simulink model "CCMMotor"  (R2022b) (continued)
== system CCMMotor == (continued)
  AirFlapPosnFrntReqForRowFirstForActrFlrLe_AirFlapPosnFrntReqForRowFirstForActrFlrLe/1 -> CCMMotor_Runnable_sys/2(AirFlapPosnFrntReqForRowFirstForActrFlrLe_AirFlapPosnFrntReqForRowFirstForActrFlrLe_read)
  AirFlapPosnFrntReqForRowFirstForActrFlrRi_AirFlapPosnFrntReqForRowFirstForActrFlrRi/1 -> CCMMotor_Runnable_sys/3(AirFlapPosnFrntReqForRowFirstForActrFlrRi_AirFlapPosnFrntReqForRowFirstForActrFlrRi_read)
  AirFlapPosnFrntReqForRowFirstForActrVentLe_AirFlapPosnFrntReqForRowFirstForActrVentLe/1 -> CCMMotor_Runnable_sys/4(AirFlapPosnFrntReqForRowFirstForActrVentLe_AirFlapPosnFrntReqForRowFirstForActrVentLe_read)
  AirFlapPosnFrntReqForRowFirstForActrVentRi_AirFlapPosnFrntReqForRowFirstForActrVentRi/1 -> CCMMotor_Runnable_sys/5(AirFlapPosnFrntReqForRowFirstForActrVentRi_AirFlapPosnFrntReqForRowFirstForActrVentRi_read)
  AirFlapPosnFrntReqForRowFirstForActrVentSideLe_AirFlapPosnFrntReqForRowFirstForActrVentSideLe/1 -> CCMMotor_Runnable_sys/6(AirFlapPosnFrntReqForRowFirstForActrVentSideLe_AirFlapPosnFrntReqForRowFirstForActrVentSideLe_read)
  AirFlapPosnFrntReqForRowFirstForActrVentSideRi_AirFlapPosnFrntReqForRowFirstForActrVentSideRi/1 -> CCMMotor_Runnable_sys/7(AirFlapPosnFrntReqForRowFirstForActrVentSideRi_AirFlapPosnFrntReqForRowFirstForActrVentSideRi_read)
  AirFlapPosnFrntReqForRowFirstForOsa_AirFlapPosnFrntReqForRowFirstForOsa/1 -> CCMMotor_Runnable_sys/8(AirFlapPosnFrntReqForRowFirstForOsa_AirFlapPosnFrntReqForRowFirstForOsa_read)
  AirFlapPosnFrntReqForRowFirstForRecirc_AirFlapPosnFrntReqForRowFirstForRecirc/1 -> CCMMotor_Runnable_sys/9(AirFlapPosnFrntReqForRowFirstForRecirc_AirFlapPosnFrntReqForRowFirstForRecirc_read)
  AirFlapPosnFrntReqForRowSecForActrTRi_AirFlapPosnFrntReqForRowSecForActrTRi/1 -> CCMMotor_Runnable_sys/10(AirFlapPosnFrntReqForRowSecForActrTRi_AirFlapPosnFrntReqForRowSecForActrTRi_read)
  PwrMdAllMtrSlp_PwrMdAllMtrSlp/1 -> CCMMotor_Runnable_sys/11(PwrMdAllMtrSlp_PwrMdAllMtrSlp_read)
  SysServCarCfgSts_SysServCarID/1 -> CCMMotor_Runnable_sys/12(SysServCarCfgSts_SysServCarID_read)
  SysServCarCfgSts_SysServCCPAQSSts/1 -> CCMMotor_Runnable_sys/13(SysServCarCfgSts_SysServCCPAQSSts_read)
  SysServCarCfgSts_SysServCCPHEXSensorSts/1 -> CCMMotor_Runnable_sys/14(SysServCarCfgSts_SysServCCPHEXSensorSts_read)
  SysServCarCfgSts_SysServCCPLin2AFUSts/1 -> CCMMotor_Runnable_sys/15(SysServCarCfgSts_SysServCCPLin2AFUSts_read)
  SysServCarCfgSts_SysServCCPLin2AIUSts/1 -> CCMMotor_Runnable_sys/16(SysServCarCfgSts_SysServCCPLin2AIUSts_read)
  SysServCarCfgSts_SysServCCPLin2CDSSts/1 -> CCMMotor_Runnable_sys/17(SysServCarCfgSts_SysServCCPLin2CDSSts_read)
  SysServCarCfgSts_SysServCCPLin2PMSISts/1 -> CCMMotor_Runnable_sys/18(SysServCarCfgSts_SysServCCPLin2PMSISts_read)
  SysServCarCfgSts_SysServCCPLin3VGASts/1 -> CCMMotor_Runnable_sys/19(SysServCarCfgSts_SysServCCPLin3VGASts_read)
  SysServCarCfgSts_SysServDUAirVentSnrSts/1 -> CCMMotor_Runnable_sys/20(SysServCarCfgSts_SysServDUAirVentSnrSts_read)
  SysServCarCfgSts_SysServDUBlowerPwmSignSts/1 -> CCMMotor_Runnable_sys/21(SysServCarCfgSts_SysServDUBlowerPwmSignSts_read)
  SysServCarCfgSts_SysServDUBlowerPwmSts/1 -> CCMMotor_Runnable_sys/22(SysServCarCfgSts_SysServDUBlowerPwmSts_read)
  SysServCarCfgSts_SysServDUDCDefrostSts/1 -> CCMMotor_Runnable_sys/23(SysServCarCfgSts_SysServDUDCDefrostSts_read)
  SysServCarCfgSts_SysServDUDCModeSts/1 -> CCMMotor_Runnable_sys/24(SysServCarCfgSts_SysServDUDCModeSts_read)
  SysServCarCfgSts_SysServDUDCRecSts/1 -> CCMMotor_Runnable_sys/25(SysServCarCfgSts_SysServDUDCRecSts_read)
  SysServCarCfgSts_SysServDUDCSModeSts/1 -> CCMMotor_Runnable_sys/26(SysServCarCfgSts_SysServDUDCSModeSts_read)
  SysServCarCfgSts_SysServDUDCSTLSts/1 -> CCMMotor_Runnable_sys/27(SysServCarCfgSts_SysServDUDCSTLSts_read)
  SysServCarCfgSts_SysServDUDCTLSts/1 -> CCMMotor_Runnable_sys/28(SysServCarCfgSts_SysServDUDCTLSts_read)
  SysServCarCfgSts_SysServDUDCTRSts/1 -> CCMMotor_Runnable_sys/29(SysServCarCfgSts_SysServDUDCTRSts_read)
  SysServCarCfgSts_SysServDUFLFootSts/1 -> CCMMotor_Runnable_sys/30(SysServCarCfgSts_SysServDUFLFootSts_read)
  SysServCarCfgSts_SysServDUFLVentSts/1 -> CCMMotor_Runnable_sys/31(SysServCarCfgSts_SysServDUFLVentSts_read)
  SysServCarCfgSts_SysServDUFRFootSts/1 -> CCMMotor_Runnable_sys/32(SysServCarCfgSts_SysServDUFRFootSts_read)
  SysServCarCfgSts_SysServDUFRVentSts/1 -> CCMMotor_Runnable_sys/33(SysServCarCfgSts_SysServDUFRVentSts_read)
  SysServCarCfgSts_SysServDUIncarMotorSts/1 -> CCMMotor_Runnable_sys/34(SysServCarCfgSts_SysServDUIncarMotorSts_read)
  SysServCarCfgSts_SysServDULin1HBMFSts/1 -> CCMMotor_Runnable_sys/35(SysServCarCfgSts_SysServDULin1HBMFSts_read)
  SysServCarCfgSts_SysServDULin4HFAFSts/1 -> CCMMotor_Runnable_sys/36(SysServCarCfgSts_SysServDULin4HFAFSts_read)
  SysServCarCfgSts_SysServDUSFLFootSts/1 -> CCMMotor_Runnable_sys/37(SysServCarCfgSts_SysServDUSFLFootSts_read)
  SysServCarCfgSts_SysServDUSFLVentSts/1 -> CCMMotor_Runnable_sys/38(SysServCarCfgSts_SysServDUSFLVentSts_read)
  SysServCarCfgSts_SysServDUSFRFootSts/1 -> CCMMotor_Runnable_sys/39(SysServCarCfgSts_SysServDUSFRFootSts_read)
  SysServCarCfgSts_SysServDUSFRVentSts/1 -> CCMMotor_Runnable_sys/40(SysServCarCfgSts_SysServDUSFRVentSts_read)
  SysServCarCfgSts_SysServDUSnsrForFCmptmtTSts/1 -> CCMMotor_Runnable_sys/41(SysServCarCfgSts_SysServDUSnsrForFCmptmtTSts_read)
  SysServCarCfgSts_SysServDUSnsrForRair/1 -> CCMMotor_Runnable_sys/42(SysServCarCfgSts_SysServDUSnsrForRair_read)
  SysServCarCfgSts_SysServDUSnsrForRCmptmtTSts/1 -> CCMMotor_Runnable_sys/43(SysServCarCfgSts_SysServDUSnsrForRCmptmtTSts_read)
  VehModMngtGlbSafe1_VehModMngtGlbSafe1/1 -> CCMMotor_Runnable_sys/44(VehModMngtGlbSafe1_VehModMngtGlbSafe1_read)
  ReqForActrCal_ReqForActrCal/1 -> CCMMotor_Runnable_sys/45(ReqForActrCal_ReqForActrCal_read)
  ActrFrntReqForRowFirstLeModDistbn_ActrFrntReqForRowFirstLeModDistbn/1 -> CCMMotor_Runnable_sys/46(ActrFrntReqForRowFirstLeModDistbn_ActrFrntReqForRowFirstLeModDistbn_read)
  ActrFrntReqForRowFirstLeModDistbn_ActrFrntReqForRowFirstLeModDistbn_IsUpdated/1 -> CCMMotor_Runnable_sys/47(ActrFrntReqForRowFirstLeModDistbn_ActrFrntReqForRowFirstLeModDistbn_IsUpdated_read)
  ActrFrntReqForRowFirstModDistbn_ActrFrntReqForRowFirstModDistbn/1 -> CCMMotor_Runnable_sys/48(ActrFrntReqForRowFirstModDistbn_ActrFrntReqForRowFirstModDistbn_read)
  ActrFrntReqForRowFirstModDistbn_ActrFrntReqForRowFirstModDistbn_IsUpdated/1 -> CCMMotor_Runnable_sys/49(ActrFrntReqForRowFirstModDistbn_ActrFrntReqForRowFirstModDistbn_IsUpdated_read)
  ActrFrntReqForRowSecModDistbnLe_ActrFrntReqForRowSecModDistbnLe/1 -> CCMMotor_Runnable_sys/50(ActrFrntReqForRowSecModDistbnLe_ActrFrntReqForRowSecModDistbnLe_read)
  ActrFrntReqForRowSecModDistbnLe_ActrFrntReqForRowSecModDistbnLe_IsUpdated/1 -> CCMMotor_Runnable_sys/51(ActrFrntReqForRowSecModDistbnLe_ActrFrntReqForRowSecModDistbnLe_IsUpdated_read)
  ActrPosnFrnReqForOsaAndRecirc_ActrPosnFrnReqForOsaAndRecirc/1 -> CCMMotor_Runnable_sys/52(ActrPosnFrnReqForOsaAndRecirc_ActrPosnFrnReqForOsaAndRecirc_read)
  ActrPosnFrnReqForOsaAndRecirc_ActrPosnFrnReqForOsaAndRecirc_IsUpdated/1 -> CCMMotor_Runnable_sys/53(ActrPosnFrnReqForOsaAndRecirc_ActrPosnFrnReqForOsaAndRecirc_IsUpdated_read)
  ActrReqForModDistbnRe_ActrReqForModDistbnRe/1 -> CCMMotor_Runnable_sys/54(ActrReqForModDistbnRe_ActrReqForModDistbnRe_read)
  ActrReqForModDistbnRe_ActrReqForModDistbnRe_IsUpdated/1 -> CCMMotor_Runnable_sys/55(ActrReqForModDistbnRe_ActrReqForModDistbnRe_IsUpdated_read)
  AirFlapPosnFrntReqForRowFirstForActrDefrst_AirFlapPosnFrntReqForRowFirstForActrDefrst/1 -> CCMMotor_Runnable_sys/56(AirFlapPosnFrntReqForRowFirstForActrDefrst_AirFlapPosnFrntReqForRowFirstForActrDefrst_read)
  AirFlapPosnFrntReqForRowFirstForActrDefrst_AirFlapPosnFrntReqForRowFirstForActrDefrst_IsUpdated/1 -> CCMMotor_Runnable_sys/57(AirFlapPosnFrntReqForRowFirstForActrDefrst_AirFlapPosnFrntReqForRowFirstForActrDefrst_IsUpdated_read)
  AirFlapPosnFrntReqForRowFirstForActrTLe_AirFlapPosnFrntReqForRowFirstForActrTLe/1 -> CCMMotor_Runnable_sys/58(AirFlapPosnFrntReqForRowFirstForActrTLe_AirFlapPosnFrntReqForRowFirstForActrTLe_read)
  AirFlapPosnFrntReqForRowFirstForActrTLe_AirFlapPosnFrntReqForRowFirstForActrTLe_IsUpdated/1 -> CCMMotor_Runnable_sys/59(AirFlapPosnFrntReqForRowFirstForActrTLe_AirFlapPosnFrntReqForRowFirstForActrTLe_IsUpdated_read)
  AirFlapPosnFrntReqForRowFirstForActrTRi_AirFlapPosnFrntReqForRowFirstForActrTRi/1 -> CCMMotor_Runnable_sys/60(AirFlapPosnFrntReqForRowFirstForActrTRi_AirFlapPosnFrntReqForRowFirstForActrTRi_read)
  AirFlapPosnFrntReqForRowFirstForActrTRi_AirFlapPosnFrntReqForRowFirstForActrTRi_IsUpdated/1 -> CCMMotor_Runnable_sys/61(AirFlapPosnFrntReqForRowFirstForActrTRi_AirFlapPosnFrntReqForRowFirstForActrTRi_IsUpdated_read)
  AirFlapPosnFrntReqForRowSecForActrTLe_AirFlapPosnFrntReqForRowSecForActrTLe/1 -> CCMMotor_Runnable_sys/62(AirFlapPosnFrntReqForRowSecForActrTLe_AirFlapPosnFrntReqForRowSecForActrTLe_read)
  AirFlapPosnFrntReqForRowSecForActrTLe_AirFlapPosnFrntReqForRowSecForActrTLe_IsUpdated/1 -> CCMMotor_Runnable_sys/63(AirFlapPosnFrntReqForRowSecForActrTLe_AirFlapPosnFrntReqForRowSecForActrTLe_IsUpdated_read)
  CarCfgForClimaCtrl_CarCfgForAirClng/1 -> CCMMotor_Runnable_sys/64(CarCfgForClimaCtrl_CarCfgForAirClng_read)
  CarCfgForClimaCtrl_CarCfgForAirClnrAdv/1 -> CCMMotor_Runnable_sys/65(CarCfgForClimaCtrl_CarCfgForAirClnrAdv_read)
  CarCfgForClimaCtrl_CarCfgForAirFrag/1 -> CCMMotor_Runnable_sys/66(CarCfgForClimaCtrl_CarCfgForAirFrag_read)
  CarCfgForClimaCtrl_CarCfgForAirMtrlSnsr/1 -> CCMMotor_Runnable_sys/67(CarCfgForClimaCtrl_CarCfgForAirMtrlSnsr_read)
  CarCfgForClimaCtrl_CarCfgForAirQly/1 -> CCMMotor_Runnable_sys/68(CarCfgForClimaCtrl_CarCfgForAirQly_read)
  CarCfgForClimaCtrl_CarCfgForAmntOfSeat/1 -> CCMMotor_Runnable_sys/69(CarCfgForClimaCtrl_CarCfgForAmntOfSeat_read)
  CarCfgForClimaCtrl_CarCfgForAutDemst/1 -> CCMMotor_Runnable_sys/70(CarCfgForClimaCtrl_CarCfgForAutDemst_read)
  CarCfgForClimaCtrl_CarCfgForAuxWtrPmp/1 -> CCMMotor_Runnable_sys/71(CarCfgForClimaCtrl_CarCfgForAuxWtrPmp_read)
  CarCfgForClimaCtrl_CarCfgForBRAND/1 -> CCMMotor_Runnable_sys/72(CarCfgForClimaCtrl_CarCfgForBRAND_read)
  CarCfgForClimaCtrl_CarCfgForBypVlvInHvac/1 -> CCMMotor_Runnable_sys/73(CarCfgForClimaCtrl_CarCfgForBypVlvInHvac_read)
  CarCfgForClimaCtrl_CarCfgForClsVlvInHvac/1 -> CCMMotor_Runnable_sys/74(CarCfgForClimaCtrl_CarCfgForClsVlvInHvac_read)
  CarCfgForClimaCtrl_CarCfgForCmprAcTyp/1 -> CCMMotor_Runnable_sys/75(CarCfgForClimaCtrl_CarCfgForCmprAcTyp_read)
  CarCfgForClimaCtrl_CarCfgForCmptmtClng/1 -> CCMMotor_Runnable_sys/76(CarCfgForClimaCtrl_CarCfgForCmptmtClng_read)
  CarCfgForClimaCtrl_CarCfgForElectricLiftingModule/1 -> CCMMotor_Runnable_sys/77(CarCfgForClimaCtrl_CarCfgForElectricLiftingModule_read)
  CarCfgForClimaCtrl_CarCfgForElecVentAdjmt/1 -> CCMMotor_Runnable_sys/78(CarCfgForClimaCtrl_CarCfgForElecVentAdjmt_read)
  CarCfgForClimaCtrl_CarCfgForExtVentnClimaCtrl/1 -> CCMMotor_Runnable_sys/79(CarCfgForClimaCtrl_CarCfgForExtVentnClimaCtrl_read)
  CarCfgForClimaCtrl_CarCfgForFlcTyp/1 -> CCMMotor_Runnable_sys/80(CarCfgForClimaCtrl_CarCfgForFlcTyp_read)
  CarCfgForClimaCtrl_CarCfgForFoldCSD/1 -> CCMMotor_Runnable_sys/81(CarCfgForClimaCtrl_CarCfgForFoldCSD_read)
  CarCfgForClimaCtrl_CarCfgForFuTyp/1 -> CCMMotor_Runnable_sys/82(CarCfgForClimaCtrl_CarCfgForFuTyp_read)
  CarCfgForClimaCtrl_CarCfgForGlassTyp/1 -> CCMMotor_Runnable_sys/83(CarCfgForClimaCtrl_CarCfgForGlassTyp_read)
  CarCfgForClimaCtrl_CarCfgForHeadRadar/1 -> CCMMotor_Runnable_sys/84(CarCfgForClimaCtrl_CarCfgForHeadRadar_read)
  CarCfgForClimaCtrl_CarCfgForHeatPmp/1 -> CCMMotor_Runnable_sys/85(CarCfgForClimaCtrl_CarCfgForHeatPmp_read)
  CarCfgForClimaCtrl_CarCfgForHeatrDurgDrvg/1 -> CCMMotor_Runnable_sys/86(CarCfgForClimaCtrl_CarCfgForHeatrDurgDrvg_read)
  CarCfgForClimaCtrl_CarCfgForHeatrTyp/1 -> CCMMotor_Runnable_sys/87(CarCfgForClimaCtrl_CarCfgForHeatrTyp_read)
  CarCfgForClimaCtrl_CarCfgForHvacFilTyp/1 -> CCMMotor_Runnable_sys/88(CarCfgForClimaCtrl_CarCfgForHvacFilTyp_read)
  CarCfgForClimaCtrl_CarCfgForHvacFlrSnsrForT/1 -> CCMMotor_Runnable_sys/89(CarCfgForClimaCtrl_CarCfgForHvacFlrSnsrForT_read)
  CarCfgForClimaCtrl_CarCfgForHvacHeatrSnsrT/1 -> CCMMotor_Runnable_sys/90(CarCfgForClimaCtrl_CarCfgForHvacHeatrSnsrT_read)
  CarCfgForClimaCtrl_CarCfgForHvacRe/1 -> CCMMotor_Runnable_sys/91(CarCfgForClimaCtrl_CarCfgForHvacRe_read)
  CarCfgForClimaCtrl_CarCfgForHvacTyp/1 -> CCMMotor_Runnable_sys/92(CarCfgForClimaCtrl_CarCfgForHvacTyp_read)
  CarCfgForClimaCtrl_CarCfgForInteriorViewCamera/1 -> CCMMotor_Runnable_sys/93(CarCfgForClimaCtrl_CarCfgForInteriorViewCamera_read)
  CarCfgForClimaCtrl_CarCfgForLifeDetection/1 -> CCMMotor_Runnable_sys/94(CarCfgForClimaCtrl_CarCfgForLifeDetection_read)
  CarCfgForClimaCtrl_CarCfgForLoVoltElecCfg/1 -> CCMMotor_Runnable_sys/95(CarCfgForClimaCtrl_CarCfgForLoVoltElecCfg_read)
  CarCfgForClimaCtrl_CarCfgForNavSysandIntnmap/1 -> CCMMotor_Runnable_sys/96(CarCfgForClimaCtrl_CarCfgForNavSysandIntnmap_read)
  CarCfgForClimaCtrl_CarCfgForNrOfDoor/1 -> CCMMotor_Runnable_sys/97(CarCfgForClimaCtrl_CarCfgForNrOfDoor_read)
  CarCfgForClimaCtrl_CarCfgForPipeInFuTank/1 -> CCMMotor_Runnable_sys/98(CarCfgForClimaCtrl_CarCfgForPipeInFuTank_read)
  CarCfgForClimaCtrl_CarCfgForPowerSeats/1 -> CCMMotor_Runnable_sys/99(CarCfgForClimaCtrl_CarCfgForPowerSeats_read)
  CarCfgForClimaCtrl_CarCfgForPreClng/1 -> CCMMotor_Runnable_sys/100(CarCfgForClimaCtrl_CarCfgForPreClng_read)
  CarCfgForClimaCtrl_CarCfgForPrkgCDSNotAvl/1 -> CCMMotor_Runnable_sys/101(CarCfgForClimaCtrl_CarCfgForPrkgCDSNotAvl_read)
  CarCfgForClimaCtrl_CarCfgForPrkgClima/1 -> CCMMotor_Runnable_sys/102(CarCfgForClimaCtrl_CarCfgForPrkgClima_read)
  CarCfgForClimaCtrl_CarCfgForPrpsnTyp/1 -> CCMMotor_Runnable_sys/103(CarCfgForClimaCtrl_CarCfgForPrpsnTyp_read)
  CarCfgForClimaCtrl_CarCfgForPTCInFrontHVAC/1 -> CCMMotor_Runnable_sys/104(CarCfgForClimaCtrl_CarCfgForPTCInFrontHVAC_read)
  CarCfgForClimaCtrl_CarCfgForRemEngStrtPreCdn/1 -> CCMMotor_Runnable_sys/105(CarCfgForClimaCtrl_CarCfgForRemEngStrtPreCdn_read)
  CarCfgForClimaCtrl_CarCfgForRoofHatch/1 -> CCMMotor_Runnable_sys/106(CarCfgForClimaCtrl_CarCfgForRoofHatch_read)
  CarCfgForClimaCtrl_CarCfgForSeatHeatgCal/1 -> CCMMotor_Runnable_sys/107(CarCfgForClimaCtrl_CarCfgForSeatHeatgCal_read)
  CarCfgForClimaCtrl_CarCfgForSeatHeatgForRowFirst/1 -> CCMMotor_Runnable_sys/108(CarCfgForClimaCtrl_CarCfgForSeatHeatgForRowFirst_read)
  CarCfgForClimaCtrl_CarCfgForSeatHeatgForRowSec/1 -> CCMMotor_Runnable_sys/109(CarCfgForClimaCtrl_CarCfgForSeatHeatgForRowSec_read)
  CarCfgForClimaCtrl_CarCfgForSeatVentnForRowFirst/1 -> CCMMotor_Runnable_sys/110(CarCfgForClimaCtrl_CarCfgForSeatVentnForRowFirst_read)
  CarCfgForClimaCtrl_CarCfgForSeatVentnForRowSec/1 -> CCMMotor_Runnable_sys/111(CarCfgForClimaCtrl_CarCfgForSeatVentnForRowSec_read)
  CarCfgForClimaCtrl_CarCfgForSecRowVentnCenter/1 -> CCMMotor_Runnable_sys/112(CarCfgForClimaCtrl_CarCfgForSecRowVentnCenter_read)
  CarCfgForClimaCtrl_CarCfgForSecRowVentnSide/1 -> CCMMotor_Runnable_sys/113(CarCfgForClimaCtrl_CarCfgForSecRowVentnSide_read)
  CarCfgForClimaCtrl_CarCfgForSmallFlowCyc/1 -> CCMMotor_Runnable_sys/114(CarCfgForClimaCtrl_CarCfgForSmallFlowCyc_read)
  CarCfgForClimaCtrl_CarCfgForSmkSnsr/1 -> CCMMotor_Runnable_sys/115(CarCfgForClimaCtrl_CarCfgForSmkSnsr_read)
  CarCfgForClimaCtrl_CarCfgForSnsrForCmptmtT/1 -> CCMMotor_Runnable_sys/116(CarCfgForClimaCtrl_CarCfgForSnsrForCmptmtT_read)
  CarCfgForClimaCtrl_CarCfgForSnsrHumPrsnt/1 -> CCMMotor_Runnable_sys/117(CarCfgForClimaCtrl_CarCfgForSnsrHumPrsnt_read)
  CarCfgForClimaCtrl_CarCfgForStartDevice/1 -> CCMMotor_Runnable_sys/118(CarCfgForClimaCtrl_CarCfgForStartDevice_read)
  CarCfgForClimaCtrl_CarCfgForSteerWhlHeatg/1 -> CCMMotor_Runnable_sys/119(CarCfgForClimaCtrl_CarCfgForSteerWhlHeatg_read)
  CarCfgForClimaCtrl_CarCfgForSteerWhlPosn/1 -> CCMMotor_Runnable_sys/120(CarCfgForClimaCtrl_CarCfgForSteerWhlPosn_read)
  CarCfgForClimaCtrl_CarCfgForSunSnsr/1 -> CCMMotor_Runnable_sys/121(CarCfgForClimaCtrl_CarCfgForSunSnsr_read)
  CarCfgForClimaCtrl_CarCfgForTelmNodTyp/1 -> CCMMotor_Runnable_sys/122(CarCfgForClimaCtrl_CarCfgForTelmNodTyp_read)
  CarCfgForClimaCtrl_CarCfgForTEMPEREDSTORAGEBOX/1 -> CCMMotor_Runnable_sys/123(CarCfgForClimaCtrl_CarCfgForTEMPEREDSTORAGEBOX_read)
  CarCfgForClimaCtrl_CarCfgForThermMngt/1 -> CCMMotor_Runnable_sys/124(CarCfgForClimaCtrl_CarCfgForThermMngt_read)
  CarCfgForClimaCtrl_CarCfgForVehTyp/1 -> CCMMotor_Runnable_sys/125(CarCfgForClimaCtrl_CarCfgForVehTyp_read)
  CarCfgForClimaCtrl_CarCfgForVentLiftMotorExiSts/1 -> CCMMotor_Runnable_sys/126(CarCfgForClimaCtrl_CarCfgForVentLiftMotorExiSts_read)
  CarCfgForClimaCtrl_CarCfgForVgaMotorExistSts/1 -> CCMMotor_Runnable_sys/127(CarCfgForClimaCtrl_CarCfgForVgaMotorExistSts_read)
  CarCfgForClimaCtrl_CarCfgForWadingAssistSystem/1 -> CCMMotor_Runnable_sys/128(CarCfgForClimaCtrl_CarCfgForWadingAssistSystem_read)
  CarCfgForClimaCtrl_CarCfgForWindHeatd/1 -> CCMMotor_Runnable_sys/129(CarCfgForClimaCtrl_CarCfgForWindHeatd_read)
  CarCfgForClimaCtrl_CarCfgWindFollowHumanMov/1 -> CCMMotor_Runnable_sys/130(CarCfgForClimaCtrl_CarCfgWindFollowHumanMov_read)
  SysServCarCfgSts_SysServCCPBGMNum/1 -> CCMMotor_Runnable_sys/131(SysServCarCfgSts_SysServCCPBGMNum_read)
  SysServCarCfgSts_SysServCCPLin2AQSSts/1 -> CCMMotor_Runnable_sys/132(SysServCarCfgSts_SysServCCPLin2AQSSts_read)
  SysServCarCfgSts_SysServDUBlowerPwmSignTypeSts/1 -> CCMMotor_Runnable_sys/133(SysServCarCfgSts_SysServDUBlowerPwmSignTypeSts_read)
  SysServCarCfgSts_SysServDUDCFLMdeSts/1 -> CCMMotor_Runnable_sys/134(SysServCarCfgSts_SysServDUDCFLMdeSts_read)
  SysServCarCfgSts_SysServDULin4HFAFStsH/1 -> CCMMotor_Runnable_sys/135(SysServCarCfgSts_SysServDULin4HFAFStsH_read)
  SysServCarCfgSts_SysServDUReBlowerPwmSignSts/1 -> CCMMotor_Runnable_sys/136(SysServCarCfgSts_SysServDUReBlowerPwmSignSts_read)
  SysServCarCfgSts_SysServDUReBlowerPwmSts/1 -> CCMMotor_Runnable_sys/137(SysServCarCfgSts_SysServDUReBlowerPwmSts_read)
  SysServCarCfgSts_SysServFrntHEXSnsrPosn/1 -> CCMMotor_Runnable_sys/138(SysServCarCfgSts_SysServFrntHEXSnsrPosn_read)
  SysServCarCfgSts_SysServLowSideRETXVSts/1 -> CCMMotor_Runnable_sys/139(SysServCarCfgSts_SysServLowSideRETXVSts_read)
  SysServCarCfgSts_SysServReHEXSnsrPosn/1 -> CCMMotor_Runnable_sys/140(SysServCarCfgSts_SysServReHEXSnsrPosn_read)
  SysServCarCfgSts_SysServRHFASts/1 -> CCMMotor_Runnable_sys/141(SysServCarCfgSts_SysServRHFASts_read)
  SysServCarCfgSts_SysServSnsrForFCmptmtTType/1 -> CCMMotor_Runnable_sys/142(SysServCarCfgSts_SysServSnsrForFCmptmtTType_read)
  VentLiftModCaliReq_VentLiftModCaliReq/1 -> CCMMotor_Runnable_sys/143(VentLiftModCaliReq_VentLiftModCaliReq_read)
  VentnDrvrLeXPsonReq_VentnDrvrLeXPsonReq/1 -> CCMMotor_Runnable_sys/144(VentnDrvrLeXPsonReq_VentnDrvrLeXPsonReq_read)
  VentnDrvrLeXPsonReq_VentnDrvrLeXPsonReq_IsUpdated/1 -> CCMMotor_Runnable_sys/145(VentnDrvrLeXPsonReq_VentnDrvrLeXPsonReq_IsUpdated_read)
  VentnDrvrLeYPsonReq_VentnDrvrLeYPsonReq/1 -> CCMMotor_Runnable_sys/146(VentnDrvrLeYPsonReq_VentnDrvrLeYPsonReq_read)
  VentnDrvrLeYPsonReq_VentnDrvrLeYPsonReq_IsUpdated/1 -> CCMMotor_Runnable_sys/147(VentnDrvrLeYPsonReq_VentnDrvrLeYPsonReq_IsUpdated_read)
  VentnDrvrRiXPsonReq_VentnDrvrRiXPsonReq/1 -> CCMMotor_Runnable_sys/148(VentnDrvrRiXPsonReq_VentnDrvrRiXPsonReq_read)
  VentnDrvrRiXPsonReq_VentnDrvrRiXPsonReq_IsUpdated/1 -> CCMMotor_Runnable_sys/149(VentnDrvrRiXPsonReq_VentnDrvrRiXPsonReq_IsUpdated_read)
  VentnDrvrRiYPsonReq_VentnDrvrRiYPsonReq/1 -> CCMMotor_Runnable_sys/150(VentnDrvrRiYPsonReq_VentnDrvrRiYPsonReq_read)
  VentnDrvrRiYPsonReq_VentnDrvrRiYPsonReq_IsUpdated/1 -> CCMMotor_Runnable_sys/151(VentnDrvrRiYPsonReq_VentnDrvrRiYPsonReq_IsUpdated_read)
  VentnLiftModPosnReq_VentnLiftModPosnReq/1 -> CCMMotor_Runnable_sys/152(VentnLiftModPosnReq_VentnLiftModPosnReq_read)
  VentnLiftModPosnReq_VentnLiftModPosnReq_IsUpdated/1 -> CCMMotor_Runnable_sys/153(VentnLiftModPosnReq_VentnLiftModPosnReq_IsUpdated_read)
  VentnPassLeXPsonReq_VentnPassLeXPsonReq/1 -> CCMMotor_Runnable_sys/154(VentnPassLeXPsonReq_VentnPassLeXPsonReq_read)
  VentnPassLeXPsonReq_VentnPassLeXPsonReq_IsUpdated/1 -> CCMMotor_Runnable_sys/155(VentnPassLeXPsonReq_VentnPassLeXPsonReq_IsUpdated_read)
  VentnPassLeYPsonReq_VentnPassLeYPsonReq/1 -> CCMMotor_Runnable_sys/156(VentnPassLeYPsonReq_VentnPassLeYPsonReq_read)
  VentnPassLeYPsonReq_VentnPassLeYPsonReq_IsUpdated/1 -> CCMMotor_Runnable_sys/157(VentnPassLeYPsonReq_VentnPassLeYPsonReq_IsUpdated_read)
  VentnPassRiXPsonReq_VentnPassRiXPsonReq/1 -> CCMMotor_Runnable_sys/158(VentnPassRiXPsonReq_VentnPassRiXPsonReq_read)
  VentnPassRiXPsonReq_VentnPassRiXPsonReq_IsUpdated/1 -> CCMMotor_Runnable_sys/159(VentnPassRiXPsonReq_VentnPassRiXPsonReq_IsUpdated_read)
  VentnPassRiYPsonReq_VentnPassRiYPsonReq/1 -> CCMMotor_Runnable_sys/160(VentnPassRiYPsonReq_VentnPassRiYPsonReq_read)
  VentnPassRiYPsonReq_VentnPassRiYPsonReq_IsUpdated/1 -> CCMMotor_Runnable_sys/161(VentnPassRiYPsonReq_VentnPassRiYPsonReq_IsUpdated_read)
  VentnSecLeXPosnReq_VentnSecLeXPosnReq/1 -> CCMMotor_Runnable_sys/162(VentnSecLeXPosnReq_VentnSecLeXPosnReq_read)
  VentnSecLeXPosnReq_VentnSecLeXPosnReq_IsUpdated/1 -> CCMMotor_Runnable_sys/163(VentnSecLeXPosnReq_VentnSecLeXPosnReq_IsUpdated_read)
  VentnSecLeYPosnReq_VentnSecLeYPosnReq/1 -> CCMMotor_Runnable_sys/164(VentnSecLeYPosnReq_VentnSecLeYPosnReq_read)
  VentnSecLeYPosnReq_VentnSecLeYPosnReq_IsUpdated/1 -> CCMMotor_Runnable_sys/165(VentnSecLeYPosnReq_VentnSecLeYPosnReq_IsUpdated_read)
  VentnSecRiXPosnReq_VentnSecRiXPosnReq/1 -> CCMMotor_Runnable_sys/166(VentnSecRiXPosnReq_VentnSecRiXPosnReq_read)
  VentnSecRiXPosnReq_VentnSecRiXPosnReq_IsUpdated/1 -> CCMMotor_Runnable_sys/167(VentnSecRiXPosnReq_VentnSecRiXPosnReq_IsUpdated_read)
  VentnSecRiYPosnReq_VentnSecRiYPosnReq/1 -> CCMMotor_Runnable_sys/168(VentnSecRiYPosnReq_VentnSecRiYPosnReq_read)
  VentnSecRiYPosnReq_VentnSecRiYPosnReq_IsUpdated/1 -> CCMMotor_Runnable_sys/169(VentnSecRiYPosnReq_VentnSecRiYPosnReq_IsUpdated_read)
  WtrBafePsonReq_WtrBafePsonReq/1 -> CCMMotor_Runnable_sys/170(WtrBafePsonReq_WtrBafePsonReq_read)
  CCMMotor_Runnable_sys/1(ActrPosnActForModDistbnRe_ActrPosnActForModDistbnRe_write) -> ActrPosnActForModDistbnRe_ActrPosnActForModDistbnRe/1
  CCMMotor_Runnable_sys/2(ActrPosnFrntActForRowFirstModDistbnLe_ActrPosnFrntActForRowFirstModDistbnLe_write) -> ActrPosnFrntActForRowFirstModDistbnLe_ActrPosnFrntActForRowFirstModDistbnLe/1
  CCMMotor_Runnable_sys/3(AirFlapFrntStsForRowFirstModDistbnLe_AirFlapFrntStsForRowFirstModDistbnLe_write) -> AirFlapFrntStsForRowFirstModDistbnLe_AirFlapFrntStsForRowFirstModDistbnLe/1
  CCMMotor_Runnable_sys/4(AirFlapStsForActModDistbnRe_AirFlapStsForActModDistbnRe_write) -> AirFlapStsForActModDistbnRe_AirFlapStsForActModDistbnRe/1
  CCMMotor_Runnable_sys/5(DriverVolThresholdLimitOfMotor_DriverVolThresholdLimitOfMotor_write) -> DriverVolThresholdLimitOfMotor_DriverVolThresholdLimitOfMotor/1
  CCMMotor_Runnable_sys/6(HvacOsaAndRecActrQfForHp_HvacOsaAndRecActrQfForHp_write) -> HvacOsaAndRecActrQfForHp_HvacOsaAndRecActrQfForHp/1
  CCMMotor_Runnable_sys/7(VentnLiftModPosnRsp_VentnLiftModPosnRsp_write) -> VentnLiftModPosnRsp_VentnLiftModPosnRsp/1
  CCMMotor_Runnable_sys/8(VentnLiftModStsRsp_VentnLiftModStsRsp_write) -> VentnLiftModStsRsp_VentnLiftModStsRsp/1
  CCMMotor_Runnable_sys/9(ActrFrntStsForRowFirstModDistbn_ActrFrntStsForRowFirstModDistbn_write) -> ActrFrntStsForRowFirstModDistbn_ActrFrntStsForRowFirstModDistbn/1
  CCMMotor_Runnable_sys/10(ActrFrntStsForRowSecModDistbnLe_ActrFrntStsForRowSecModDistbnLe_write) -> ActrFrntStsForRowSecModDistbnLe_ActrFrntStsForRowSecModDistbnLe/1
  CCMMotor_Runnable_sys/11(ActrFrntStsForRowSecModDistbnRi_ActrFrntStsForRowSecModDistbnRi_write) -> ActrFrntStsForRowSecModDistbnRi_ActrFrntStsForRowSecModDistbnRi/1
  CCMMotor_Runnable_sys/12(ActrPosnFrntActForOsaAndRecirc_ActrPosnFrntActForOsaAndRecirc_write) -> ActrPosnFrntActForOsaAndRecirc_ActrPosnFrntActForOsaAndRecirc/1
  CCMMotor_Runnable_sys/13(ActrPosnFrntActForRowFirstModDistbn_ActrPosnFrntActForRowFirstModDistbn_write) -> ActrPosnFrntActForRowFirstModDistbn_ActrPosnFrntActForRowFirstModDistbn/1
  CCMMotor_Runnable_sys/14(ActrPosnFrntActForRowSecModDistbnLe_ActrPosnFrntActForRowSecModDistbnLe_write) -> ActrPosnFrntActForRowSecModDistbnLe_ActrPosnFrntActForRowSecModDistbnLe/1
  CCMMotor_Runnable_sys/15(ActrPosnFrntActForRowSecModDistbnRi_ActrPosnFrntActForRowSecModDistbnRi_write) -> ActrPosnFrntActForRowSecModDistbnRi_ActrPosnFrntActForRowSecModDistbnRi/1
  CCMMotor_Runnable_sys/16(AirFlapFrntStsForRowFirstForActrDefrst_AirFlapFrntStsForRowFirstForActrDefrst_write) -> AirFlapFrntStsForRowFirstForActrDefrst_AirFlapFrntStsForRowFirstForActrDefrst/1
  CCMMotor_Runnable_sys/17(AirFlapFrntStsForRowFirstForActrFlrLe_AirFlapFrntStsForRowFirstForActrFlrLe_write) -> AirFlapFrntStsForRowFirstForActrFlrLe_AirFlapFrntStsForRowFirstForActrFlrLe/1
  CCMMotor_Runnable_sys/18(AirFlapFrntStsForRowFirstForActrFlrRi_AirFlapFrntStsForRowFirstForActrFlrRi_write) -> AirFlapFrntStsForRowFirstForActrFlrRi_AirFlapFrntStsForRowFirstForActrFlrRi/1
  CCMMotor_Runnable_sys/19(AirFlapFrntStsForRowFirstForActrTLe_AirFlapFrntStsForRowFirstForActrTLe_write) -> AirFlapFrntStsForRowFirstForActrTLe_AirFlapFrntStsForRowFirstForActrTLe/1
  CCMMotor_Runnable_sys/20(AirFlapFrntStsForRowFirstForActrTRi_AirFlapFrntStsForRowFirstForActrTRi_write) -> AirFlapFrntStsForRowFirstForActrTRi_AirFlapFrntStsForRowFirstForActrTRi/1
  CCMMotor_Runnable_sys/21(AirFlapFrntStsForRowFirstForActrVentLe_AirFlapFrntStsForRowFirstForActrVentLe_write) -> AirFlapFrntStsForRowFirstForActrVentLe_AirFlapFrntStsForRowFirstForActrVentLe/1
  CCMMotor_Runnable_sys/22(AirFlapFrntStsForRowFirstForActrVentRi_AirFlapFrntStsForRowFirstForActrVentRi_write) -> AirFlapFrntStsForRowFirstForActrVentRi_AirFlapFrntStsForRowFirstForActrVentRi/1
  CCMMotor_Runnable_sys/23(AirFlapFrntStsForRowFirstForActrVentSideLe_AirFlapFrntStsForRowFirstForActrVentSideLe_write) -> AirFlapFrntStsForRowFirstForActrVentSideLe_AirFlapFrntStsForRowFirstForActrVentSideLe/1
  CCMMotor_Runnable_sys/24(AirFlapFrntStsForRowFirstForActrVentSideRi_AirFlapFrntStsForRowFirstForActrVentSideRi_write) -> AirFlapFrntStsForRowFirstForActrVentSideRi_AirFlapFrntStsForRowFirstForActrVentSideRi/1
  CCMMotor_Runnable_sys/25(AirFlapFrntStsForRowFirstForOsa_AirFlapFrntStsForRowFirstForOsa_write) -> AirFlapFrntStsForRowFirstForOsa_AirFlapFrntStsForRowFirstForOsa/1
  CCMMotor_Runnable_sys/26(AirFlapFrntStsForRowFirstForOsaAndRecirc_AirFlapFrntStsForRowFirstForOsaAndRecirc_write) -> AirFlapFrntStsForRowFirstForOsaAndRecirc_AirFlapFrntStsForRowFirstForOsaAndRecirc/1
  CCMMotor_Runnable_sys/27(AirFlapFrntStsForRowFirstForRecirc_AirFlapFrntStsForRowFirstForRecirc_write) -> AirFlapFrntStsForRowFirstForRecirc_AirFlapFrntStsForRowFirstForRecirc/1
  CCMMotor_Runnable_sys/28(AirFlapFrntStsForRowSecForActrTLe_AirFlapFrntStsForRowSecForActrTLe_write) -> AirFlapFrntStsForRowSecForActrTLe_AirFlapFrntStsForRowSecForActrTLe/1
  CCMMotor_Runnable_sys/29(AirFlapFrntStsForRowSecForActrTRi_AirFlapFrntStsForRowSecForActrTRi_write) -> AirFlapFrntStsForRowSecForActrTRi_AirFlapFrntStsForRowSecForActrTRi/1
  CCMMotor_Runnable_sys/30(AirFlapPosnFrntActForRowFirstForActrDefrst_AirFlapPosnFrntActForRowFirstForActrDefrst_write) -> AirFlapPosnFrntActForRowFirstForActrDefrst_AirFlapPosnFrntActForRowFirstForActrDefrst/1
  CCMMotor_Runnable_sys/31(AirFlapPosnFrntActForRowFirstForActrFlrLe_AirFlapPosnFrntActForRowFirstForActrFlrLe_write) -> AirFlapPosnFrntActForRowFirstForActrFlrLe_AirFlapPosnFrntActForRowFirstForActrFlrLe/1
  CCMMotor_Runnable_sys/32(AirFlapPosnFrntActForRowFirstForActrFlrRi_AirFlapPosnFrntActForRowFirstForActrFlrRi_write) -> AirFlapPosnFrntActForRowFirstForActrFlrRi_AirFlapPosnFrntActForRowFirstForActrFlrRi/1
  CCMMotor_Runnable_sys/33(AirFlapPosnFrntActForRowFirstForActrTLe_AirFlapPosnFrntActForRowFirstForActrTLe_write) -> AirFlapPosnFrntActForRowFirstForActrTLe_AirFlapPosnFrntActForRowFirstForActrTLe/1
  CCMMotor_Runnable_sys/34(AirFlapPosnFrntActForRowFirstForActrTRi_AirFlapPosnFrntActForRowFirstForActrTRi_write) -> AirFlapPosnFrntActForRowFirstForActrTRi_AirFlapPosnFrntActForRowFirstForActrTRi/1
  CCMMotor_Runnable_sys/35(AirFlapPosnFrntActForRowFirstForActrVentLe_AirFlapPosnFrntActForRowFirstForActrVentLe_write) -> AirFlapPosnFrntActForRowFirstForActrVentLe_AirFlapPosnFrntActForRowFirstForActrVentLe/1
  CCMMotor_Runnable_sys/36(AirFlapPosnFrntActForRowFirstForActrVentRi_AirFlapPosnFrntActForRowFirstForActrVentRi_write) -> AirFlapPosnFrntActForRowFirstForActrVentRi_AirFlapPosnFrntActForRowFirstForActrVentRi/1
  CCMMotor_Runnable_sys/37(AirFlapPosnFrntActForRowFirstForActrVentSideLe_AirFlapPosnFrntActForRowFirstForActrVentSideLe_write) -> AirFlapPosnFrntActForRowFirstForActrVentSideLe_AirFlapPosnFrntActForRowFirstForActrVentSideLe/1
  CCMMotor_Runnable_sys/38(AirFlapPosnFrntActForRowFirstForActrVentSideRi_AirFlapPosnFrntActForRowFirstForActrVentSideRi_write) -> AirFlapPosnFrntActForRowFirstForActrVentSideRi_AirFlapPosnFrntActForRowFirstForActrVentSideRi/1
  CCMMotor_Runnable_sys/39(AirFlapPosnFrntActForRowFirstForOsa_AirFlapPosnFrntActForRowFirstForOsa_write) -> AirFlapPosnFrntActForRowFirstForOsa_AirFlapPosnFrntActForRowFirstForOsa/1
  CCMMotor_Runnable_sys/40(AirFlapPosnFrntActForRowFirstForRecirc_AirFlapPosnFrntActForRowFirstForRecirc_write) -> AirFlapPosnFrntActForRowFirstForRecirc_AirFlapPosnFrntActForRowFirstForRecirc/1
  CCMMotor_Runnable_sys/41(AirFlapPosnFrntActForRowSecForActrTLe_AirFlapPosnFrntActForRowSecForActrTLe_write) -> AirFlapPosnFrntActForRowSecForActrTLe_AirFlapPosnFrntActForRowSecForActrTLe/1
  CCMMotor_Runnable_sys/42(AirFlapPosnFrntActForRowSecForActrTRi_AirFlapPosnFrntActForRowSecForActrTRi_write) -> AirFlapPosnFrntActForRowSecForActrTRi_AirFlapPosnFrntActForRowSecForActrTRi/1
  CCMMotor_Runnable_sys/43(CcmElecAirDirCrtlReqDrvrLePosX_CcmElecAirDirCrtlReqDrvrLePosX_write) -> CcmElecAirDirCrtlReqDrvrLePosX_CcmElecAirDirCrtlReqDrvrLePosX/1
  CCMMotor_Runnable_sys/44(CcmElecAirDirCrtlReqDrvrLePosY_CcmElecAirDirCrtlReqDrvrLePosY_write) -> CcmElecAirDirCrtlReqDrvrLePosY_CcmElecAirDirCrtlReqDrvrLePosY/1
  CCMMotor_Runnable_sys/45(CcmElecAirDirCrtlReqDrvrRiPosX_CcmElecAirDirCrtlReqDrvrRiPosX_write) -> CcmElecAirDirCrtlReqDrvrRiPosX_CcmElecAirDirCrtlReqDrvrRiPosX/1
  CCMMotor_Runnable_sys/46(CcmElecAirDirCrtlReqDrvrRiPosY_CcmElecAirDirCrtlReqDrvrRiPosY_write) -> CcmElecAirDirCrtlReqDrvrRiPosY_CcmElecAirDirCrtlReqDrvrRiPosY/1
  CCMMotor_Runnable_sys/47(CcmElecAirDirCrtlReqPassLePosX_CcmElecAirDirCrtlReqPassLePosX_write) -> CcmElecAirDirCrtlReqPassLePosX_CcmElecAirDirCrtlReqPassLePosX/1
  CCMMotor_Runnable_sys/48(CcmElecAirDirCrtlReqPassLePosY_CcmElecAirDirCrtlReqPassLePosY_write) -> CcmElecAirDirCrtlReqPassLePosY_CcmElecAirDirCrtlReqPassLePosY/1
  CCMMotor_Runnable_sys/49(CcmElecAirDirCrtlReqPassRiPosX_CcmElecAirDirCrtlReqPassRiPosX_write) -> CcmElecAirDirCrtlReqPassRiPosX_CcmElecAirDirCrtlReqPassRiPosX/1
  CCMMotor_Runnable_sys/50(CcmElecAirDirCrtlReqPassRiPosY_CcmElecAirDirCrtlReqPassRiPosY_write) -> CcmElecAirDirCrtlReqPassRiPosY_CcmElecAirDirCrtlReqPassRiPosY/1
  CCMMotor_Runnable_sys/51(FrntVGAerror_FrntVGAerror_write) -> FrntVGAerror_FrntVGAerror/1
  CCMMotor_Runnable_sys/52(RearVGAerror_RearVGAerror_write) -> RearVGAerror_RearVGAerror/1
  CCMMotor_Runnable_sys/53(VentnDrvrLeXStsRsp_VentnDrvrLeXStsRsp_write) -> VentnDrvrLeXStsRsp_VentnDrvrLeXStsRsp/1
  CCMMotor_Runnable_sys/54(VentnDrvrLeYStsRsp_VentnDrvrLeYStsRsp_write) -> VentnDrvrLeYStsRsp_VentnDrvrLeYStsRsp/1
  CCMMotor_Runnable_sys/55(VentnDrvrRiXStsRsp_VentnDrvrRiXStsRsp_write) -> VentnDrvrRiXStsRsp_VentnDrvrRiXStsRsp/1
  CCMMotor_Runnable_sys/56(VentnDrvrRiYStsRsp_VentnDrvrRiYStsRsp_write) -> VentnDrvrRiYStsRsp_VentnDrvrRiYStsRsp/1
  CCMMotor_Runnable_sys/57(VentnPassLeXStsRsp_VentnPassLeXStsRsp_write) -> VentnPassLeXStsRsp_VentnPassLeXStsRsp/1
  CCMMotor_Runnable_sys/58(VentnPassLeYStsRsp_VentnPassLeYStsRsp_write) -> VentnPassLeYStsRsp_VentnPassLeYStsRsp/1
  CCMMotor_Runnable_sys/59(VentnPassRiXStsRsp_VentnPassRiXStsRsp_write) -> VentnPassRiXStsRsp_VentnPassRiXStsRsp/1
  CCMMotor_Runnable_sys/60(VentnPassRiYStsRsp_VentnPassRiYStsRsp_write) -> VentnPassRiYStsRsp_VentnPassRiYStsRsp/1
  CCMMotor_Runnable_sys/61(VentnSecLeXStsRsp_VentnSecLeXStsRsp_write) -> VentnSecLeXStsRsp_VentnSecLeXStsRsp/1
  CCMMotor_Runnable_sys/62(VentnSecLeYStsRsp_VentnSecLeYStsRsp_write) -> VentnSecLeYStsRsp_VentnSecLeYStsRsp/1
  CCMMotor_Runnable_sys/63(VentnSecRiXStsRsp_VentnSecRiXStsRsp_write) -> VentnSecRiXStsRsp_VentnSecRiXStsRsp/1
  CCMMotor_Runnable_sys/64(VentnSecRiYStsRsp_VentnSecRiYStsRsp_write) -> VentnSecRiYStsRsp_VentnSecRiYStsRsp/1
  BswLifeCycle_LifeCycle/1 -> CCMMotor_Runnable_sys/171(BswLifeCycle_LifeCycle_read)
  CCMMotor_Runnable/1 -> CCMMotor_Runnable_sys/Trigger
